simulink model "fft_direct_example"  (Simulink 7.6)
settings: Solver=ode45  StartTime=0.0  StopTime=10.0  RelTol=1e-3
libraries: xbsIndex_r4 x4688, casper_library x192
(part 34 of 39)

== system fft_direct_example/butterfly3_0/twiddle/dsp48e_complex_mult ==
  b_re : Inport
  b_im : Inport  Port=2
  w_re : Inport  Port=3
  w_im : Inport  Port=4
  DSP48E : xbsIndex_r4/DSP48E  use_a=Direct from A Port  use_b=Direct from B Port  pattern_from_creg=off  pattern='000000000000'  mask_from_creg=off  pattern_mask='3FFFFFFFFFFF'  reset_preg=off  sel_rounding_mask=Select mask  use_op=off  use_creg=off  rst_all=off  en_all=off  use_pcin=on  use_carrycascin=off  use_multsignin=off  use_carryout=off  use_patterndetect=off  use_patternbdetect=off  use_overflow=off  use_underflow=off  use_acout=off  use_bcout=off  use_pcout=off  use_multsignout=off  use_carrycascout=off  pipeline_a=2  pipeline_b=2  pipeline_acout=1  pipeline_bcout=1  pipeline_c=on  pipeline_p=on  pipeline_mult=on  pipeline_opmode=on  pipeline_alumode=on  pipeline_carryin=on  pipeline_carryinsel=on  rst_a=off  rst_b=off  rst_c=off  rst_mult=off  rst_p=off  rst_carry_in=off  rst_alumode=off  rst_ctrl=off  en_a1=off  en_a2=off  en_b1=off  en_b2=off  en_c=off  en_mult=off  en_p=off  en_carry_in=off  en_alumode=off  en_multcarryin=off  en_ctrl=off  use_synth_model=off  addsub_mode=ONE48  xl_useadderonly=off  block_type=dsp48e
      settings: block_version=10.1.3
  DSP48E1 : xbsIndex_r4/DSP48E  use_a=Direct from A Port  use_b=Direct from B Port  pattern_from_creg=off  pattern='000000000000'  mask_from_creg=off  pattern_mask='3FFFFFFFFFFF'  reset_preg=off  sel_rounding_mask=Select mask  use_op=off  use_creg=off  rst_all=off  en_all=off  use_pcin=off  use_carrycascin=off  use_multsignin=off  use_carryout=off  use_patterndetect=off  use_patternbdetect=off  use_overflow=off  use_underflow=off  use_acout=off  use_bcout=off  use_pcout=on  use_multsignout=off  use_carrycascout=off  pipeline_a=1  pipeline_b=1  pipeline_acout=1  pipeline_bcout=1  pipeline_c=on  pipeline_p=on  pipeline_mult=on  pipeline_opmode=on  pipeline_alumode=on  pipeline_carryin=on  pipeline_carryinsel=on  rst_a=off  rst_b=off  rst_c=off  rst_mult=off  rst_p=off  rst_carry_in=off  rst_alumode=off  rst_ctrl=off  en_a1=off  en_a2=off  en_b1=off  en_b2=off  en_c=off  en_mult=off  en_p=off  en_carry_in=off  en_alumode=off  en_multcarryin=off  en_ctrl=off  use_synth_model=off  addsub_mode=ONE48  xl_useadderonly=off  block_type=dsp48e
      settings: block_version=10.1.3
  DSP48E2 : xbsIndex_r4/DSP48E  use_a=Direct from A Port  use_b=Direct from B Port  pattern_from_creg=off  pattern='000000000000'  mask_from_creg=off  pattern_mask='3FFFFFFFFFFF'  reset_preg=off  sel_rounding_mask=Select mask  use_op=off  use_creg=off  rst_all=off  en_all=off  use_pcin=on  use_carrycascin=off  use_multsignin=off  use_carryout=off  use_patterndetect=off  use_patternbdetect=off  use_overflow=off  use_underflow=off  use_acout=off  use_bcout=off  use_pcout=off  use_multsignout=off  use_carrycascout=off  pipeline_a=2  pipeline_b=2  pipeline_acout=1  pipeline_bcout=1  pipeline_c=on  pipeline_p=on  pipeline_mult=on  pipeline_opmode=on  pipeline_alumode=on  pipeline_carryin=on  pipeline_carryinsel=on  rst_a=off  rst_b=off  rst_c=off  rst_mult=off  rst_p=off  rst_carry_in=off  rst_alumode=off  rst_ctrl=off  en_a1=off  en_a2=off  en_b1=off  en_b2=off  en_c=off  en_mult=off  en_p=off  en_carry_in=off  en_alumode=off  en_multcarryin=off  en_ctrl=off  use_synth_model=off  addsub_mode=ONE48  xl_useadderonly=off  block_type=dsp48e
      settings: block_version=10.1.3
  DSP48E3 : xbsIndex_r4/DSP48E  use_a=Direct from A Port  use_b=Direct from B Port  pattern_from_creg=off  pattern='000000000000'  mask_from_creg=off  pattern_mask='3FFFFFFFFFFF'  reset_preg=off  sel_rounding_mask=Select mask  use_op=off  use_creg=off  rst_all=off  en_all=off  use_pcin=off  use_carrycascin=off  use_multsignin=off  use_carryout=off  use_patterndetect=off  use_patternbdetect=off  use_overflow=off  use_underflow=off  use_acout=off  use_bcout=off  use_pcout=on  use_multsignout=off  use_carrycascout=off  pipeline_a=1  pipeline_b=1  pipeline_acout=1  pipeline_bcout=1  pipeline_c=on  pipeline_p=on  pipeline_mult=on  pipeline_opmode=on  pipeline_alumode=on  pipeline_carryin=on  pipeline_carryinsel=on  rst_a=off  rst_b=off  rst_c=off  rst_mult=off  rst_p=off  rst_carry_in=off  rst_alumode=off  rst_ctrl=off  en_a1=off  en_a2=off  en_b1=off  en_b2=off  en_c=off  en_mult=off  en_p=off  en_carry_in=off  en_alumode=off  en_multcarryin=off  en_ctrl=off  use_synth_model=off  addsub_mode=ONE48  xl_useadderonly=off  block_type=dsp48e
      settings: block_version=10.1.3
  Terminator : Terminator
  Terminator1 : Terminator
  alucode : xbsIndex_r4/Constant  arith_type=Unsigned  const=0  n_bits=4  bin_pt=0  explicit_period=on  period=1  equ=P=C  opselect=C  inp2=PCIN>>17  opr=+  inp1=P  carry=CIN  dbl_ovrd=off  block_type=constant
  alucode1 : xbsIndex_r4/Constant  arith_type=Unsigned  const=0  n_bits=4  bin_pt=0  explicit_period=on  period=1  equ=P=C  opselect=C  inp2=PCIN>>17  opr=+  inp1=P  carry=CIN  dbl_ovrd=off  block_type=constant
  alucode2 : xbsIndex_r4/Constant  arith_type=Unsigned  const=3  n_bits=4  bin_pt=0  explicit_period=on  period=1  equ=P=C  opselect=C  inp2=PCIN>>17  opr=+  inp1=P  carry=CIN  dbl_ovrd=off  block_type=constant
  alucode3 : xbsIndex_r4/Constant  arith_type=Unsigned  const=0  n_bits=4  bin_pt=0  explicit_period=on  period=1  equ=P=C  opselect=C  inp2=PCIN>>17  opr=+  inp1=P  carry=CIN  dbl_ovrd=off  block_type=constant
  carryin{,1..3} (4 alike) : xbsIndex_r4/Constant  arith_type=Unsigned  const=0  n_bits=1  bin_pt=0  explicit_period=on  period=1  equ=P=C  opselect=C  inp2=PCIN>>17  opr=+  inp1=P  carry=CIN  dbl_ovrd=off  block_type=constant
  carryinsel{,1..3} (4 alike) : xbsIndex_r4/Constant  arith_type=Unsigned  const=0  n_bits=3  bin_pt=0  explicit_period=on  period=1  equ=P=C  opselect=C  inp2=PCIN>>17  opr=+  inp1=P  carry=CIN  dbl_ovrd=off  block_type=constant
  opcode : xbsIndex_r4/Constant  arith_type=Unsigned  const=21  n_bits=7  bin_pt=0  explicit_period=on  period=1  equ=P=C  opselect=C  inp2=PCIN>>17  opr=+  inp1=P  carry=CIN  dbl_ovrd=off  block_type=constant
  opcode1 : xbsIndex_r4/Constant  arith_type=Unsigned  const=5  n_bits=7  bin_pt=0  explicit_period=on  period=1  equ=P=C  opselect=C  inp2=PCIN>>17  opr=+  inp1=P  carry=CIN  dbl_ovrd=off  block_type=constant
  opcode2 : xbsIndex_r4/Constant  arith_type=Unsigned  const=21  n_bits=7  bin_pt=0  explicit_period=on  period=1  equ=P=C  opselect=C  inp2=PCIN>>17  opr=+  inp1=P  carry=CIN  dbl_ovrd=off  block_type=constant
  opcode3 : xbsIndex_r4/Constant  arith_type=Unsigned  const=5  n_bits=7  bin_pt=0  explicit_period=on  period=1  equ=P=C  opselect=C  inp2=PCIN>>17  opr=+  inp1=P  carry=CIN  dbl_ovrd=off  block_type=constant
  bw_re : Outport
  bw_im : Outport  Port=2
  wires:
  [w_re] -> DSP48E/1
  [b_im] -> DSP48E/2
  b_im/1 -> [b_im]
  w_re/1 -> [w_re]
  [b_re] -> DSP48E1/2
  b_re/1 -> [b_re]
  [w_im] -> DSP48E2/1
  [w_im] -> DSP48E1/1
  w_im/1 -> [w_im]
  DSP48E3/2 -> DSP48E2/3
  [b_im] -> DSP48E2/2
  [b_re] -> DSP48E3/2
  [w_re] -> DSP48E3/1
  DSP48E2/1 -> bw_re/1
  DSP48E3/1 -> Terminator1/1
  carryinsel2/1 -> DSP48E2/7
  carryin2/1 -> DSP48E2/6
  alucode2/1 -> DSP48E2/5
  opcode2/1 -> DSP48E2/4
  opcode3/1 -> DSP48E3/3
  alucode3/1 -> DSP48E3/4
  carryin3/1 -> DSP48E3/5
  carryinsel3/1 -> DSP48E3/6
  DSP48E1/2 -> DSP48E/3
  carryinsel1/1 -> DSP48E1/6
  carryin1/1 -> DSP48E1/5
  alucode1/1 -> DSP48E1/4
  opcode1/1 -> DSP48E1/3
  opcode/1 -> DSP48E/4
  alucode/1 -> DSP48E/5
  carryin/1 -> DSP48E/6
  carryinsel/1 -> DSP48E/7
  DSP48E1/1 -> Terminator/1
  DSP48E/1 -> bw_im/1

== system fft_direct_example/butterfly3_1/twiddle/coeff_gen ==
  rst : Inport
  Counter : xbsIndex_r4/Counter  cnt_type=Free Running  cnt_to=Inf  operation=Up  start_count=0  cnt_by_val=1  arith_type=Unsigned  n_bits=6  bin_pt=0  load_pin=off  rst=on  en=off  explicit_period=on  period=1  dbl_ovrd=off  use_behavioral_HDL=off  implementation=Fabric  use_rpm=off  block_type=counter
  ROM : xbsIndex_r4/ROM  depth=64  initVector="[1                     0.9999237060546875                    0.99970245361328125                    0.99932098388671875                     0.9987945556640625                    0.99811553955078125                    0.99729156494140625                    0.99631500244140625                    0.99518585205078125                    0.99390411376953125                     0.9924774169921875                       0.99090576171875                    0.98917388916015625                           0.9873046875                     0.9852752685546875                     0.9831085205078125                    0.98078155517578125                     0.9783172607421875                    0.97570037841796875                    0.97293853759765625                       0.97003173828125                       0.96697998046875                      0.963775634765625                     0.9604339599609375                      0.956939697265625                       0.95330810546875                    0.94953155517578125                    0.94561004638671875                     0.9415435791015625                    0.93733978271484375                    0.93299102783203125                    0.92850494384765625                    0.92388153076171875                     0.9191131591796875                    0.91420745849609375                     0.9091644287109375                       0.90399169921875                    0.89867401123046875                    0.89322662353515625                    0.88764190673828125                    0.88191986083984375                      0.876068115234375                      0.870086669921875                    0.86397552490234375                       0.85772705078125                    0.85135650634765625                    0.84485626220703125                      0.838226318359375                     0.8314666748046875                    0.82459259033203125                     0.8175811767578125                      0.810455322265625                     0.8032073974609375                       0.79583740234375                     0.7883453369140625                    0.78073883056640625                       0.77301025390625                      0.765167236328125                    0.75720977783203125                    0.74913787841796875                     0.7409515380859375                     0.7326507568359375                    0.72425079345703125                      0.715728759765625]"  distributed_mem=Block RAM  rst=off  init_reg=0  en=off  latency=3  arith_type="Signed  (2's comp)"  n_bits=18  bin_pt=17  dbl_ovrd=off  optimize=Area  use_rpm=on  block_type=sprom
      settings: block_version=10.1.3
  ROM1 : xbsIndex_r4/ROM  depth=64  initVector="[0                      -0.01226806640625                   -0.02454376220703125                      -0.03680419921875                   -0.04906463623046875                   -0.06131744384765625                    -0.0735626220703125                    -0.0858001708984375                   -0.09801483154296875                   -0.11022186279296875                   -0.12241363525390625                      -0.13458251953125                        -0.146728515625                    -0.1588592529296875                      -0.17095947265625                   -0.18303680419921875                   -0.19509124755859375                   -0.20711517333984375                    -0.2191009521484375                   -0.23105621337890625                      -0.24298095703125                    -0.2548675537109375                   -0.26671600341796875                    -0.2785186767578125                        -0.290283203125                   -0.30200958251953125                   -0.31368255615234375                   -0.32530975341796875                   -0.33689117431640625                     -0.348419189453125                     -0.359893798828125                   -0.37131500244140625                   -0.38268280029296875                   -0.39398956298828125                     -0.405242919921875                    -0.4164276123046875                   -0.42755889892578125                    -0.4386138916015625                   -0.44960784912109375                     -0.460540771484375                   -0.47139739990234375                   -0.48218536376953125                   -0.49289703369140625                       -0.5035400390625                      -0.51409912109375                   -0.52458953857421875                   -0.53499603271484375                   -0.54532623291015625                     -0.555572509765625                      -0.56573486328125                       -0.5758056640625                    -0.5858001708984375                   -0.59569549560546875                    -0.6055145263671875                           -0.615234375                    -0.6248626708984375                   -0.63439178466796875                     -0.643829345703125                   -0.65317535400390625                      -0.66241455078125                   -0.67156219482421875                      -0.68060302734375                   -0.68953704833984375                    -0.6983795166015625]"  distributed_mem=Block RAM  rst=off  init_reg=0  en=off  latency=3  arith_type="Signed  (2's comp)"  n_bits=18  bin_pt=17  dbl_ovrd=off  optimize=Area  use_rpm=on  block_type=sprom
      settings: block_version=10.1.3
  Slice : xbsIndex_r4/Slice  nbits=6  boolean_output=off  mode=Upper Bit Location + Width  bit1=0  base1=MSB of Input  bit0=0  base0=LSB of Input  dbl_ovrd=off  block_type=slice
      settings: infoedit="Extracts a given range of bits from each input sample and presents it at the output.  The output type is ordinarily unsigned with binary point at zero, but can be Boolean when the slice is one bit wide.<P><P>Hardware notes: In hardware this block costs nothing."  block_version=10.1.3
  ri_to_c : casper_library/Misc/ri_to_c  SystemSampleTime=-1
  w : Outport
  wires:
  ROM1/1 -> ri_to_c/2
  ROM/1 -> ri_to_c/1
  Slice/1 -> ROM1/1, ROM/1
  Counter/1 -> Slice/1
  rst/1 -> Counter/1
  ri_to_c/1 -> w/1

== system fft_direct_example/butterfly3_1/twiddle/dsp48e_complex_mult ==
  b_re : Inport
  b_im : Inport  Port=2
  w_re : Inport  Port=3
  w_im : Inport  Port=4
  DSP48E : xbsIndex_r4/DSP48E  use_a=Direct from A Port  use_b=Direct from B Port  pattern_from_creg=off  pattern='000000000000'  mask_from_creg=off  pattern_mask='3FFFFFFFFFFF'  reset_preg=off  sel_rounding_mask=Select mask  use_op=off  use_creg=off  rst_all=off  en_all=off  use_pcin=on  use_carrycascin=off  use_multsignin=off  use_carryout=off  use_patterndetect=off  use_patternbdetect=off  use_overflow=off  use_underflow=off  use_acout=off  use_bcout=off  use_pcout=off  use_multsignout=off  use_carrycascout=off  pipeline_a=2  pipeline_b=2  pipeline_acout=1  pipeline_bcout=1  pipeline_c=on  pipeline_p=on  pipeline_mult=on  pipeline_opmode=on  pipeline_alumode=on  pipeline_carryin=on  pipeline_carryinsel=on  rst_a=off  rst_b=off  rst_c=off  rst_mult=off  rst_p=off  rst_carry_in=off  rst_alumode=off  rst_ctrl=off  en_a1=off  en_a2=off  en_b1=off  en_b2=off  en_c=off  en_mult=off  en_p=off  en_carry_in=off  en_alumode=off  en_multcarryin=off  en_ctrl=off  use_synth_model=off  addsub_mode=ONE48  xl_useadderonly=off  block_type=dsp48e
      settings: block_version=10.1.3
  DSP48E1 : xbsIndex_r4/DSP48E  use_a=Direct from A Port  use_b=Direct from B Port  pattern_from_creg=off  pattern='000000000000'  mask_from_creg=off  pattern_mask='3FFFFFFFFFFF'  reset_preg=off  sel_rounding_mask=Select mask  use_op=off  use_creg=off  rst_all=off  en_all=off  use_pcin=off  use_carrycascin=off  use_multsignin=off  use_carryout=off  use_patterndetect=off  use_patternbdetect=off  use_overflow=off  use_underflow=off  use_acout=off  use_bcout=off  use_pcout=on  use_multsignout=off  use_carrycascout=off  pipeline_a=1  pipeline_b=1  pipeline_acout=1  pipeline_bcout=1  pipeline_c=on  pipeline_p=on  pipeline_mult=on  pipeline_opmode=on  pipeline_alumode=on  pipeline_carryin=on  pipeline_carryinsel=on  rst_a=off  rst_b=off  rst_c=off  rst_mult=off  rst_p=off  rst_carry_in=off  rst_alumode=off  rst_ctrl=off  en_a1=off  en_a2=off  en_b1=off  en_b2=off  en_c=off  en_mult=off  en_p=off  en_carry_in=off  en_alumode=off  en_multcarryin=off  en_ctrl=off  use_synth_model=off  addsub_mode=ONE48  xl_useadderonly=off  block_type=dsp48e
      settings: block_version=10.1.3
  DSP48E2 : xbsIndex_r4/DSP48E  use_a=Direct from A Port  use_b=Direct from B Port  pattern_from_creg=off  pattern='000000000000'  mask_from_creg=off  pattern_mask='3FFFFFFFFFFF'  reset_preg=off  sel_rounding_mask=Select mask  use_op=off  use_creg=off  rst_all=off  en_all=off  use_pcin=on  use_carrycascin=off  use_multsignin=off  use_carryout=off  use_patterndetect=off  use_patternbdetect=off  use_overflow=off  use_underflow=off  use_acout=off  use_bcout=off  use_pcout=off  use_multsignout=off  use_carrycascout=off  pipeline_a=2  pipeline_b=2  pipeline_acout=1  pipeline_bcout=1  pipeline_c=on  pipeline_p=on  pipeline_mult=on  pipeline_opmode=on  pipeline_alumode=on  pipeline_carryin=on  pipeline_carryinsel=on  rst_a=off  rst_b=off  rst_c=off  rst_mult=off  rst_p=off  rst_carry_in=off  rst_alumode=off  rst_ctrl=off  en_a1=off  en_a2=off  en_b1=off  en_b2=off  en_c=off  en_mult=off  en_p=off  en_carry_in=off  en_alumode=off  en_multcarryin=off  en_ctrl=off  use_synth_model=off  addsub_mode=ONE48  xl_useadderonly=off  block_type=dsp48e
      settings: block_version=10.1.3
  DSP48E3 : xbsIndex_r4/DSP48E  use_a=Direct from A Port  use_b=Direct from B Port  pattern_from_creg=off  pattern='000000000000'  mask_from_creg=off  pattern_mask='3FFFFFFFFFFF'  reset_preg=off  sel_rounding_mask=Select mask  use_op=off  use_creg=off  rst_all=off  en_all=off  use_pcin=off  use_carrycascin=off  use_multsignin=off  use_carryout=off  use_patterndetect=off  use_patternbdetect=off  use_overflow=off  use_underflow=off  use_acout=off  use_bcout=off  use_pcout=on  use_multsignout=off  use_carrycascout=off  pipeline_a=1  pipeline_b=1  pipeline_acout=1  pipeline_bcout=1  pipeline_c=on  pipeline_p=on  pipeline_mult=on  pipeline_opmode=on  pipeline_alumode=on  pipeline_carryin=on  pipeline_carryinsel=on  rst_a=off  rst_b=off  rst_c=off  rst_mult=off  rst_p=off  rst_carry_in=off  rst_alumode=off  rst_ctrl=off  en_a1=off  en_a2=off  en_b1=off  en_b2=off  en_c=off  en_mult=off  en_p=off  en_carry_in=off  en_alumode=off  en_multcarryin=off  en_ctrl=off  use_synth_model=off  addsub_mode=ONE48  xl_useadderonly=off  block_type=dsp48e
      settings: block_version=10.1.3
  Terminator : Terminator
  Terminator1 : Terminator
  alucode : xbsIndex_r4/Constant  arith_type=Unsigned  const=0  n_bits=4  bin_pt=0  explicit_period=on  period=1  equ=P=C  opselect=C  inp2=PCIN>>17  opr=+  inp1=P  carry=CIN  dbl_ovrd=off  block_type=constant
  alucode1 : xbsIndex_r4/Constant  arith_type=Unsigned  const=0  n_bits=4  bin_pt=0  explicit_period=on  period=1  equ=P=C  opselect=C  inp2=PCIN>>17  opr=+  inp1=P  carry=CIN  dbl_ovrd=off  block_type=constant
  alucode2 : xbsIndex_r4/Constant  arith_type=Unsigned  const=3  n_bits=4  bin_pt=0  explicit_period=on  period=1  equ=P=C  opselect=C  inp2=PCIN>>17  opr=+  inp1=P  carry=CIN  dbl_ovrd=off  block_type=constant
  alucode3 : xbsIndex_r4/Constant  arith_type=Unsigned  const=0  n_bits=4  bin_pt=0  explicit_period=on  period=1  equ=P=C  opselect=C  inp2=PCIN>>17  opr=+  inp1=P  carry=CIN  dbl_ovrd=off  block_type=constant
  carryin{,1..3} (4 alike) : xbsIndex_r4/Constant  arith_type=Unsigned  const=0  n_bits=1  bin_pt=0  explicit_period=on  period=1  equ=P=C  opselect=C  inp2=PCIN>>17  opr=+  inp1=P  carry=CIN  dbl_ovrd=off  block_type=constant
  carryinsel{,1..3} (4 alike) : xbsIndex_r4/Constant  arith_type=Unsigned  const=0  n_bits=3  bin_pt=0  explicit_period=on  period=1  equ=P=C  opselect=C  inp2=PCIN>>17  opr=+  inp1=P  carry=CIN  dbl_ovrd=off  block_type=constant
  opcode : xbsIndex_r4/Constant  arith_type=Unsigned  const=21  n_bits=7  bin_pt=0  explicit_period=on  period=1  equ=P=C  opselect=C  inp2=PCIN>>17  opr=+  inp1=P  carry=CIN  dbl_ovrd=off  block_type=constant
  opcode1 : xbsIndex_r4/Constant  arith_type=Unsigned  const=5  n_bits=7  bin_pt=0  explicit_period=on  period=1  equ=P=C  opselect=C  inp2=PCIN>>17  opr=+  inp1=P  carry=CIN  dbl_ovrd=off  block_type=constant
  opcode2 : xbsIndex_r4/Constant  arith_type=Unsigned  const=21  n_bits=7  bin_pt=0  explicit_period=on  period=1  equ=P=C  opselect=C  inp2=PCIN>>17  opr=+  inp1=P  carry=CIN  dbl_ovrd=off  block_type=constant
  opcode3 : xbsIndex_r4/Constant  arith_type=Unsigned  const=5  n_bits=7  bin_pt=0  explicit_period=on  period=1  equ=P=C  opselect=C  inp2=PCIN>>17  opr=+  inp1=P  carry=CIN  dbl_ovrd=off  block_type=constant
  bw_re : Outport
  bw_im : Outport  Port=2
  wires:
  [w_re] -> DSP48E/1
  [b_im] -> DSP48E/2
  b_im/1 -> [b_im]
  w_re/1 -> [w_re]
  [b_re] -> DSP48E1/2
  b_re/1 -> [b_re]
  [w_im] -> DSP48E2/1
  [w_im] -> DSP48E1/1
  w_im/1 -> [w_im]
  DSP48E3/2 -> DSP48E2/3
  [b_im] -> DSP48E2/2
  [b_re] -> DSP48E3/2
  [w_re] -> DSP48E3/1
  DSP48E2/1 -> bw_re/1
  DSP48E3/1 -> Terminator1/1
  carryinsel2/1 -> DSP48E2/7
  carryin2/1 -> DSP48E2/6
  alucode2/1 -> DSP48E2/5
  opcode2/1 -> DSP48E2/4
  opcode3/1 -> DSP48E3/3
  alucode3/1 -> DSP48E3/4
  carryin3/1 -> DSP48E3/5
  carryinsel3/1 -> DSP48E3/6
  DSP48E1/2 -> DSP48E/3
  carryinsel1/1 -> DSP48E1/6
  carryin1/1 -> DSP48E1/5
  alucode1/1 -> DSP48E1/4
  opcode1/1 -> DSP48E1/3
  opcode/1 -> DSP48E/4
  alucode/1 -> DSP48E/5
  carryin/1 -> DSP48E/6
  carryinsel/1 -> DSP48E/7
  DSP48E1/1 -> Terminator/1
  DSP48E/1 -> bw_im/1

== system fft_direct_example/butterfly3_2/twiddle/coeff_gen ==
  rst : Inport
  Counter : xbsIndex_r4/Counter  cnt_type=Free Running  cnt_to=Inf  operation=Up  start_count=0  cnt_by_val=1  arith_type=Unsigned  n_bits=6  bin_pt=0  load_pin=off  rst=on  en=off  explicit_period=on  period=1  dbl_ovrd=off  use_behavioral_HDL=off  implementation=Fabric  use_rpm=off  block_type=counter
  ROM : xbsIndex_r4/ROM  depth=64  initVector="[0                      -0.01226806640625                   -0.02454376220703125                      -0.03680419921875                   -0.04906463623046875                   -0.06131744384765625                    -0.0735626220703125                    -0.0858001708984375                   -0.09801483154296875                   -0.11022186279296875                   -0.12241363525390625                      -0.13458251953125                        -0.146728515625                    -0.1588592529296875                      -0.17095947265625                   -0.18303680419921875                   -0.19509124755859375                   -0.20711517333984375                    -0.2191009521484375                   -0.23105621337890625                      -0.24298095703125                    -0.2548675537109375                   -0.26671600341796875                    -0.2785186767578125                        -0.290283203125                   -0.30200958251953125                   -0.31368255615234375                   -0.32530975341796875                   -0.33689117431640625                     -0.348419189453125                     -0.359893798828125                   -0.37131500244140625                   -0.38268280029296875                   -0.39398956298828125                     -0.405242919921875                    -0.4164276123046875                   -0.42755889892578125                    -0.4386138916015625                   -0.44960784912109375                     -0.460540771484375                   -0.47139739990234375                   -0.48218536376953125                   -0.49289703369140625                       -0.5035400390625                      -0.51409912109375                   -0.52458953857421875                   -0.53499603271484375                   -0.54532623291015625                     -0.555572509765625                      -0.56573486328125                       -0.5758056640625                    -0.5858001708984375                   -0.59569549560546875                    -0.6055145263671875                           -0.615234375                    -0.6248626708984375                   -0.63439178466796875                     -0.643829345703125                   -0.65317535400390625                      -0.66241455078125                   -0.67156219482421875                      -0.68060302734375                   -0.68953704833984375                    -0.6983795166015625]"  distributed_mem=Block RAM  rst=off  init_reg=0  en=off  latency=3  arith_type="Signed  (2's comp)"  n_bits=18  bin_pt=17  dbl_ovrd=off  optimize=Area  use_rpm=on  block_type=sprom
      settings: block_version=10.1.3
  ROM1 : xbsIndex_r4/ROM  depth=64  initVector="[-1                    -0.9999237060546875                   -0.99970245361328125                   -0.99932098388671875                    -0.9987945556640625                   -0.99811553955078125                   -0.99729156494140625                   -0.99631500244140625                   -0.99518585205078125                   -0.99390411376953125                    -0.9924774169921875                      -0.99090576171875                   -0.98917388916015625                          -0.9873046875                    -0.9852752685546875                    -0.9831085205078125                   -0.98078155517578125                    -0.9783172607421875                   -0.97570037841796875                   -0.97293853759765625                      -0.97003173828125                      -0.96697998046875                     -0.963775634765625                    -0.9604339599609375                     -0.956939697265625                      -0.95330810546875                   -0.94953155517578125                   -0.94561004638671875                    -0.9415435791015625                   -0.93733978271484375                   -0.93299102783203125                   -0.92850494384765625                   -0.92388153076171875                    -0.9191131591796875                   -0.91420745849609375                    -0.9091644287109375                      -0.90399169921875                   -0.89867401123046875                   -0.89322662353515625                   -0.88764190673828125                   -0.88191986083984375                     -0.876068115234375                     -0.870086669921875                   -0.86397552490234375                      -0.85772705078125                   -0.85135650634765625                   -0.84485626220703125                     -0.838226318359375                    -0.8314666748046875                   -0.82459259033203125                    -0.8175811767578125                     -0.810455322265625                    -0.8032073974609375                      -0.79583740234375                    -0.7883453369140625                   -0.78073883056640625                      -0.77301025390625                     -0.765167236328125                   -0.75720977783203125                   -0.74913787841796875                    -0.7409515380859375                    -0.7326507568359375                   -0.72425079345703125                     -0.715728759765625]"  distributed_mem=Block RAM  rst=off  init_reg=0  en=off  latency=3  arith_type="Signed  (2's comp)"  n_bits=18  bin_pt=17  dbl_ovrd=off  optimize=Area  use_rpm=on  block_type=sprom
      settings: block_version=10.1.3
  Slice : xbsIndex_r4/Slice  nbits=6  boolean_output=off  mode=Upper Bit Location + Width  bit1=0  base1=MSB of Input  bit0=0  base0=LSB of Input  dbl_ovrd=off  block_type=slice
      settings: infoedit="Extracts a given range of bits from each input sample and presents it at the output.  The output type is ordinarily unsigned with binary point at zero, but can be Boolean when the slice is one bit wide.<P><P>Hardware notes: In hardware this block costs nothing."  block_version=10.1.3
  ri_to_c : casper_library/Misc/ri_to_c  SystemSampleTime=-1
  w : Outport
  wires:
  ROM1/1 -> ri_to_c/2
  ROM/1 -> ri_to_c/1
  Slice/1 -> ROM1/1, ROM/1
  Counter/1 -> Slice/1
  rst/1 -> Counter/1
  ri_to_c/1 -> w/1

== system fft_direct_example/butterfly3_2/twiddle/dsp48e_complex_mult ==
  b_re : Inport
  b_im : Inport  Port=2
  w_re : Inport  Port=3
  w_im : Inport  Port=4
  DSP48E : xbsIndex_r4/DSP48E  use_a=Direct from A Port  use_b=Direct from B Port  pattern_from_creg=off  pattern='000000000000'  mask_from_creg=off  pattern_mask='3FFFFFFFFFFF'  reset_preg=off  sel_rounding_mask=Select mask  use_op=off  use_creg=off  rst_all=off  en_all=off  use_pcin=on  use_carrycascin=off  use_multsignin=off  use_carryout=off  use_patterndetect=off  use_patternbdetect=off  use_overflow=off  use_underflow=off  use_acout=off  use_bcout=off  use_pcout=off  use_multsignout=off  use_carrycascout=off  pipeline_a=2  pipeline_b=2  pipeline_acout=1  pipeline_bcout=1  pipeline_c=on  pipeline_p=on  pipeline_mult=on  pipeline_opmode=on  pipeline_alumode=on  pipeline_carryin=on  pipeline_carryinsel=on  rst_a=off  rst_b=off  rst_c=off  rst_mult=off  rst_p=off  rst_carry_in=off  rst_alumode=off  rst_ctrl=off  en_a1=off  en_a2=off  en_b1=off  en_b2=off  en_c=off  en_mult=off  en_p=off  en_carry_in=off  en_alumode=off  en_multcarryin=off  en_ctrl=off  use_synth_model=off  addsub_mode=ONE48  xl_useadderonly=off  block_type=dsp48e
      settings: block_version=10.1.3
  DSP48E1 : xbsIndex_r4/DSP48E  use_a=Direct from A Port  use_b=Direct from B Port  pattern_from_creg=off  pattern='000000000000'  mask_from_creg=off  pattern_mask='3FFFFFFFFFFF'  reset_preg=off  sel_rounding_mask=Select mask  use_op=off  use_creg=off  rst_all=off  en_all=off  use_pcin=off  use_carrycascin=off  use_multsignin=off  use_carryout=off  use_patterndetect=off  use_patternbdetect=off  use_overflow=off  use_underflow=off  use_acout=off  use_bcout=off  use_pcout=on  use_multsignout=off  use_carrycascout=off  pipeline_a=1  pipeline_b=1  pipeline_acout=1  pipeline_bcout=1  pipeline_c=on  pipeline_p=on  pipeline_mult=on  pipeline_opmode=on  pipeline_alumode=on  pipeline_carryin=on  pipeline_carryinsel=on  rst_a=off  rst_b=off  rst_c=off  rst_mult=off  rst_p=off  rst_carry_in=off  rst_alumode=off  rst_ctrl=off  en_a1=off  en_a2=off  en_b1=off  en_b2=off  en_c=off  en_mult=off  en_p=off  en_carry_in=off  en_alumode=off  en_multcarryin=off  en_ctrl=off  use_synth_model=off  addsub_mode=ONE48  xl_useadderonly=off  block_type=dsp48e
      settings: block_version=10.1.3
  DSP48E2 : xbsIndex_r4/DSP48E  use_a=Direct from A Port  use_b=Direct from B Port  pattern_from_creg=off  pattern='000000000000'  mask_from_creg=off  pattern_mask='3FFFFFFFFFFF'  reset_preg=off  sel_rounding_mask=Select mask  use_op=off  use_creg=off  rst_all=off  en_all=off  use_pcin=on  use_carrycascin=off  use_multsignin=off  use_carryout=off  use_patterndetect=off  use_patternbdetect=off  use_overflow=off  use_underflow=off  use_acout=off  use_bcout=off  use_pcout=off  use_multsignout=off  use_carrycascout=off  pipeline_a=2  pipeline_b=2  pipeline_acout=1  pipeline_bcout=1  pipeline_c=on  pipeline_p=on  pipeline_mult=on  pipeline_opmode=on  pipeline_alumode=on  pipeline_carryin=on  pipeline_carryinsel=on  rst_a=off  rst_b=off  rst_c=off  rst_mult=off  rst_p=off  rst_carry_in=off  rst_alumode=off  rst_ctrl=off  en_a1=off  en_a2=off  en_b1=off  en_b2=off  en_c=off  en_mult=off  en_p=off  en_carry_in=off  en_alumode=off  en_multcarryin=off  en_ctrl=off  use_synth_model=off  addsub_mode=ONE48  xl_useadderonly=off  block_type=dsp48e
      settings: block_version=10.1.3
  DSP48E3 : xbsIndex_r4/DSP48E  use_a=Direct from A Port  use_b=Direct from B Port  pattern_from_creg=off  pattern='000000000000'  mask_from_creg=off  pattern_mask='3FFFFFFFFFFF'  reset_preg=off  sel_rounding_mask=Select mask  use_op=off  use_creg=off  rst_all=off  en_all=off  use_pcin=off  use_carrycascin=off  use_multsignin=off  use_carryout=off  use_patterndetect=off  use_patternbdetect=off  use_overflow=off  use_underflow=off  use_acout=off  use_bcout=off  use_pcout=on  use_multsignout=off  use_carrycascout=off  pipeline_a=1  pipeline_b=1  pipeline_acout=1  pipeline_bcout=1  pipeline_c=on  pipeline_p=on  pipeline_mult=on  pipeline_opmode=on  pipeline_alumode=on  pipeline_carryin=on  pipeline_carryinsel=on  rst_a=off  rst_b=off  rst_c=off  rst_mult=off  rst_p=off  rst_carry_in=off  rst_alumode=off  rst_ctrl=off  en_a1=off  en_a2=off  en_b1=off  en_b2=off  en_c=off  en_mult=off  en_p=off  en_carry_in=off  en_alumode=off  en_multcarryin=off  en_ctrl=off  use_synth_model=off  addsub_mode=ONE48  xl_useadderonly=off  block_type=dsp48e
      settings: block_version=10.1.3
  Terminator : Terminator
  Terminator1 : Terminator
  alucode : xbsIndex_r4/Constant  arith_type=Unsigned  const=0  n_bits=4  bin_pt=0  explicit_period=on  period=1  equ=P=C  opselect=C  inp2=PCIN>>17  opr=+  inp1=P  carry=CIN  dbl_ovrd=off  block_type=constant
  alucode1 : xbsIndex_r4/Constant  arith_type=Unsigned  const=0  n_bits=4  bin_pt=0  explicit_period=on  period=1  equ=P=C  opselect=C  inp2=PCIN>>17  opr=+  inp1=P  carry=CIN  dbl_ovrd=off  block_type=constant
  alucode2 : xbsIndex_r4/Constant  arith_type=Unsigned  const=3  n_bits=4  bin_pt=0  explicit_period=on  period=1  equ=P=C  opselect=C  inp2=PCIN>>17  opr=+  inp1=P  carry=CIN  dbl_ovrd=off  block_type=constant
  alucode3 : xbsIndex_r4/Constant  arith_type=Unsigned  const=0  n_bits=4  bin_pt=0  explicit_period=on  period=1  equ=P=C  opselect=C  inp2=PCIN>>17  opr=+  inp1=P  carry=CIN  dbl_ovrd=off  block_type=constant
  carryin{,1..3} (4 alike) : xbsIndex_r4/Constant  arith_type=Unsigned  const=0  n_bits=1  bin_pt=0  explicit_period=on  period=1  equ=P=C  opselect=C  inp2=PCIN>>17  opr=+  inp1=P  carry=CIN  dbl_ovrd=off  block_type=constant
  carryinsel{,1..3} (4 alike) : xbsIndex_r4/Constant  arith_type=Unsigned  const=0  n_bits=3  bin_pt=0  explicit_period=on  period=1  equ=P=C  opselect=C  inp2=PCIN>>17  opr=+  inp1=P  carry=CIN  dbl_ovrd=off  block_type=constant
  opcode : xbsIndex_r4/Constant  arith_type=Unsigned  const=21  n_bits=7  bin_pt=0  explicit_period=on  period=1  equ=P=C  opselect=C  inp2=PCIN>>17  opr=+  inp1=P  carry=CIN  dbl_ovrd=off  block_type=constant
  opcode1 : xbsIndex_r4/Constant  arith_type=Unsigned  const=5  n_bits=7  bin_pt=0  explicit_period=on  period=1  equ=P=C  opselect=C  inp2=PCIN>>17  opr=+  inp1=P  carry=CIN  dbl_ovrd=off  block_type=constant
  opcode2 : xbsIndex_r4/Constant  arith_type=Unsigned  const=21  n_bits=7  bin_pt=0  explicit_period=on  period=1  equ=P=C  opselect=C  inp2=PCIN>>17  opr=+  inp1=P  carry=CIN  dbl_ovrd=off  block_type=constant
  opcode3 : xbsIndex_r4/Constant  arith_type=Unsigned  const=5  n_bits=7  bin_pt=0  explicit_period=on  period=1  equ=P=C  opselect=C  inp2=PCIN>>17  opr=+  inp1=P  carry=CIN  dbl_ovrd=off  block_type=constant
  bw_re : Outport
  bw_im : Outport  Port=2
  wires:
  [w_re] -> DSP48E/1
  [b_im] -> DSP48E/2
  b_im/1 -> [b_im]
  w_re/1 -> [w_re]
  [b_re] -> DSP48E1/2
  b_re/1 -> [b_re]
  [w_im] -> DSP48E2/1
  [w_im] -> DSP48E1/1
  w_im/1 -> [w_im]
  DSP48E3/2 -> DSP48E2/3
  [b_im] -> DSP48E2/2
  [b_re] -> DSP48E3/2
  [w_re] -> DSP48E3/1
  DSP48E2/1 -> bw_re/1
  DSP48E3/1 -> Terminator1/1
  carryinsel2/1 -> DSP48E2/7
  carryin2/1 -> DSP48E2/6
  alucode2/1 -> DSP48E2/5
  opcode2/1 -> DSP48E2/4
  opcode3/1 -> DSP48E3/3
  alucode3/1 -> DSP48E3/4
  carryin3/1 -> DSP48E3/5
  carryinsel3/1 -> DSP48E3/6
  DSP48E1/2 -> DSP48E/3
  carryinsel1/1 -> DSP48E1/6
  carryin1/1 -> DSP48E1/5
  alucode1/1 -> DSP48E1/4
  opcode1/1 -> DSP48E1/3
  opcode/1 -> DSP48E/4
  alucode/1 -> DSP48E/5
  carryin/1 -> DSP48E/6
  carryinsel/1 -> DSP48E/7
  DSP48E1/1 -> Terminator/1
  DSP48E/1 -> bw_im/1

== system fft_direct_example/butterfly3_3/twiddle/coeff_gen ==
  rst : Inport
  Counter : xbsIndex_r4/Counter  cnt_type=Free Running  cnt_to=Inf  operation=Up  start_count=0  cnt_by_val=1  arith_type=Unsigned  n_bits=6  bin_pt=0  load_pin=off  rst=on  en=off  explicit_period=on  period=1  dbl_ovrd=off  use_behavioral_HDL=off  implementation=Fabric  use_rpm=off  block_type=counter
  ROM : xbsIndex_r4/ROM  depth=64  initVector="[0                      -0.01226806640625                   -0.02454376220703125                      -0.03680419921875                   -0.04906463623046875                   -0.06131744384765625                    -0.0735626220703125                    -0.0858001708984375                   -0.09801483154296875                   -0.11022186279296875                   -0.12241363525390625                      -0.13458251953125                        -0.146728515625                    -0.1588592529296875                      -0.17095947265625                   -0.18303680419921875                   -0.19509124755859375                   -0.20711517333984375                    -0.2191009521484375                   -0.23105621337890625                      -0.24298095703125                    -0.2548675537109375                   -0.26671600341796875                    -0.2785186767578125                        -0.290283203125                   -0.30200958251953125                   -0.31368255615234375                   -0.32530975341796875                   -0.33689117431640625                     -0.348419189453125                     -0.359893798828125                   -0.37131500244140625                   -0.38268280029296875                   -0.39398956298828125                     -0.405242919921875                    -0.4164276123046875                   -0.42755889892578125                    -0.4386138916015625                   -0.44960784912109375                     -0.460540771484375                   -0.47139739990234375                   -0.48218536376953125                   -0.49289703369140625                       -0.5035400390625                      -0.51409912109375                   -0.52458953857421875                   -0.53499603271484375                   -0.54532623291015625                     -0.555572509765625                      -0.56573486328125                       -0.5758056640625                    -0.5858001708984375                   -0.59569549560546875                    -0.6055145263671875                           -0.615234375                    -0.6248626708984375                   -0.63439178466796875                     -0.643829345703125                   -0.65317535400390625                      -0.66241455078125                   -0.67156219482421875                      -0.68060302734375                   -0.68953704833984375                    -0.6983795166015625]"  distributed_mem=Block RAM  rst=off  init_reg=0  en=off  latency=3  arith_type="Signed  (2's comp)"  n_bits=18  bin_pt=17  dbl_ovrd=off  optimize=Area  use_rpm=on  block_type=sprom
      settings: block_version=10.1.3
  ROM1 : xbsIndex_r4/ROM  depth=64  initVector="[-1                    -0.9999237060546875                   -0.99970245361328125                   -0.99932098388671875                    -0.9987945556640625                   -0.99811553955078125                   -0.99729156494140625                   -0.99631500244140625                   -0.99518585205078125                   -0.99390411376953125                    -0.9924774169921875                      -0.99090576171875                   -0.98917388916015625                          -0.9873046875                    -0.9852752685546875                    -0.9831085205078125                   -0.98078155517578125                    -0.9783172607421875                   -0.97570037841796875                   -0.97293853759765625                      -0.97003173828125                      -0.96697998046875                     -0.963775634765625                    -0.9604339599609375                     -0.956939697265625                      -0.95330810546875                   -0.94953155517578125                   -0.94561004638671875                    -0.9415435791015625                   -0.93733978271484375                   -0.93299102783203125                   -0.92850494384765625                   -0.92388153076171875                    -0.9191131591796875                   -0.91420745849609375                    -0.9091644287109375                      -0.90399169921875                   -0.89867401123046875                   -0.89322662353515625                   -0.88764190673828125                   -0.88191986083984375                     -0.876068115234375                     -0.870086669921875                   -0.86397552490234375                      -0.85772705078125                   -0.85135650634765625                   -0.84485626220703125                     -0.838226318359375                    -0.8314666748046875                   -0.82459259033203125                    -0.8175811767578125                     -0.810455322265625                    -0.8032073974609375                      -0.79583740234375                    -0.7883453369140625                   -0.78073883056640625                      -0.77301025390625                     -0.765167236328125                   -0.75720977783203125                   -0.74913787841796875                    -0.7409515380859375                    -0.7326507568359375                   -0.72425079345703125                     -0.715728759765625]"  distributed_mem=Block RAM  rst=off  init_reg=0  en=off  latency=3  arith_type="Signed  (2's comp)"  n_bits=18  bin_pt=17  dbl_ovrd=off  optimize=Area  use_rpm=on  block_type=sprom
      settings: block_version=10.1.3
  Slice : xbsIndex_r4/Slice  nbits=6  boolean_output=off  mode=Upper Bit Location + Width  bit1=0  base1=MSB of Input  bit0=0  base0=LSB of Input  dbl_ovrd=off  block_type=slice
      settings: infoedit="Extracts a given range of bits from each input sample and presents it at the output.  The output type is ordinarily unsigned with binary point at zero, but can be Boolean when the slice is one bit wide.<P><P>Hardware notes: In hardware this block costs nothing."  block_version=10.1.3
  ri_to_c : casper_library/Misc/ri_to_c  SystemSampleTime=-1
  w : Outport
  wires:
  ROM1/1 -> ri_to_c/2
  ROM/1 -> ri_to_c/1
  Slice/1 -> ROM1/1, ROM/1
  Counter/1 -> Slice/1
  rst/1 -> Counter/1
  ri_to_c/1 -> w/1
